simulink library "LibrarySimulinkSASv2016a"  (R2018a)
settings: Solver=ode45  StartTime=0.0  StopTime=10.0  RelTol=1e-3
undefined symbols (not defined in the file or in its folder): IdB, IdL, IdM, shmControl, ts
libraries: LibrarySimulinkSAS x15, $bdroot x1
(part 2 of 4)

== system LibrarySimulinkSASv2016a/Ardrone Parrot ==
  Pitch : Inport
  Roll : Inport  Port=2
  Gaz : Inport  Port=3
  Yaw rate : Inport  Port=4
  Generic Shared Memory Block : S-Function  FunctionName=matlabGenericSHM  Parameters=nombre,dispositivo,vecE,vecS,tipoE,tipoS,ts  SFunctionDeploymentMode=off  EnableBusSupport=off  mask(Generic Share Memory block V2.0){nombre=Dev, dispositivo=1, vecE=[ 1 1 1 1], vecS=[20], tipoE=['d' 'd' 'd'  'd' ], tipoS=['d'], ts=0}
      mask prompts: nombre "Share Memory base name"; dispositivo "Device Number"; vecE "Sizes input vector"; vecS "Sizes output vector"; tipoE "Data Types Inputs"; tipoS "Data Types Outputs"; ts "Sample Time"
      mask help:
      | This block allows Simulink communication with external processes through shared memory. It works on Unix and Windows.
      | More information in Help.
      mask Help:
      | Share Memory base name (nombre): It's the base string of share memory ej 'robot'
      | Device Number (dispositivo): integer that identifies the device between multiple in use
      | Two shared memorys are created with names:
      | "%name_entrada_%dispositivo" for the device input (writing)
      | "%name_salida_%dispositivo" for the device output (reading)
      | Sizes input vector (vecE)  : array that specify the number of inputs (array dimension) and the inputs size (array values)
      | Sizes output vector  (vecS): array that specify the number of outputs (array dimension) and the outputs size (array values)
      | Data types input(tipoE): char array that specify each input types
      | Data types output (tipoS): char array that specify each output types
      | Allowed types are:
      | ('f'=float, 'd'=double, 'i'=int32, 'c'=char, 'C'= unsigned char, 'I'=unsigned int)
      | Example.
      | Suppose the following parameters:
      | nombre='exampleMemory'
      | dispositivo=10
      | vecE=[3 2 1]
      | vecS=[6 5 7 8]
      | tipoE=['f' 'I' 'c']
      | tipoS=['C' 'i' 'd' 'd']
      | 
      | Two Share Memories are created with names:
      | "exampleMemory_entrada_10" (block input)
      | "exampleMemory_salida_10"  (block output)
      | 3 inputs are created:
      |  1° Dimension 3, type "unsigned char"
      |  2° Dimension 2, type "int"
      |  3° Dimension 1, type "char"
      | 4 outputs are created:
      |  1° Dimension 6, type "float"
      |  2° Dimension 5, type "unsigned int"
      |  3° Dimension 7, type "double"
      |  3° Dimension 8, type "double"
      mask Display: image('shmem.jpg')
  Simple Share Memory Block : S-Function  FunctionName=simpleSHM  Parameters=nombre,dispositivo,vecE,vecS,tipoE,tipoS  SFunctionDeploymentMode=off  EnableBusSupport=off  mask(Share Memory Block version 2.0){nombre='ARDRONE_video', dispositivo=1, vecE=[], vecS=[320*240*3], tipoE=[], tipoS=['C']}
      mask prompts: nombre "Share Memory base name"; dispositivo "Device Number"; vecE "Sizes input vector"; vecS "Sizes output vector"; tipoE "Data Types Inputs"; tipoS "Data Types Outputs"
      mask help: This block allows Simulink communication with external processes through shared memory. It works on Unix and Windows
      mask Help:
      | Share Memory base name (nombre): It's the base string of share memory ej 'robot'
      | Device Number (dispositivo): integer that identifies the device between multiple in use
      | Only one Share memory will be created, so you should specify only the inputs or outputs but not both
      | One shared memorys is created with name: "%name_%dispositivo"
      | 
      | Sizes input vector (vecE)  : array that specify the number of inputs (array dimension) and the inputs size (array values)
      | Sizes output vector  (vecS): array that specify the number of outputs (array dimension) and the outputs size (array values)
      | Data types input(tipoE): char array that specify each input types
      | Data types output (tipoS): char array that specify each output types
      | Allowed types are:
      | ('f'=float, 'd'=double, 'i'=int32, 'c'=char, 'C'= unsigned char, 'I'=unsigned int)
      | 
      | Example.
      | Suppose the following parameters:
      | nombre='exampleMemory'
      | dispositivo=10
      | vecE=[3 2 1]
      | vecS=[]
      | tipoE=['f' 'I' 'c']
      | tipoS=[]
      | 
      | One Share Memorie is created with name:
      | "exampleMemory_10" (block input)
      | 
      | 3 inputs are created:
      |  1° Dimension 3, type "unsigned char"
      |  2° Dimension 2, type "int"
      |  3° Dimension 1, type "char"
      mask Display: image('memoryChip.png')
  toRGB image1 : Subsystem  mask{ResX=320, ResY=240}
      mask prompts: ResX "Resolucion Camara X"; ResY "Resolucion Camara Y"
      mask Display:
      | port_label('output', 1, 'RGB')
      | port_label('input', 1, 'videoIN')
  Ardrone State : Outport
  Ardrone Video : Outport  Port=2
  wires:
  Generic Shared Memory Block/1 -> Ardrone State/1
  Yaw rate/1 -> Generic Shared Memory Block/4
  Gaz/1 -> Generic Shared Memory Block/3
  Pitch/1 -> Generic Shared Memory Block/1
  Roll/1 -> Generic Shared Memory Block/2
  toRGB image1/1(Out1) -> Ardrone Video/1
  Simple Share Memory Block/1 -> toRGB image1/1(In1)

== system LibrarySimulinkSASv2016a/Arduino+motor shield ==
  Run : Inport
  U1 : Inport  Port=2
  U2 : Inport  Port=3
  Generic Shared Memory Block : S-Function  FunctionName=matlabGenericSHM  Parameters=nombre,dispositivo,vecE,vecS,tipoE,tipoS,ts  SFunctionDeploymentMode=off  EnableBusSupport=off  mask(Generic Share Memory block V2.0){nombre='ARDUINO', dispositivo=dev, vecE=[1 1 1 ], vecS=[1 1 1 1 1 1 1 1 1], tipoE=['f' 'f' 'f' ], tipoS=['f' 'f' 'f' 'f' 'f' 'f' 'f' 'f' 'f'], ts=0}
      mask prompts: nombre "Share Memory base name"; dispositivo "Device Number"; vecE "Sizes input vector"; vecS "Sizes output vector"; tipoE "Data Types Inputs"; tipoS "Data Types Outputs"; ts "Sample Time"
      mask help:
      | This block allows Simulink communication with external processes through shared memory. It works on Unix and Windows.
      | More information in Help.
      mask Help:
      | Share Memory base name (nombre): It's the base string of share memory ej 'robot'
      | Device Number (dispositivo): integer that identifies the device between multiple in use
      | Two shared memorys are created with names:
      | "%name_entrada_%dispositivo" for the device input (writing)
      | "%name_salida_%dispositivo" for the device output (reading)
      | Sizes input vector (vecE)  : array that specify the number of inputs (array dimension) and the inputs size (array values)
      | Sizes output vector  (vecS): array that specify the number of outputs (array dimension) and the outputs size (array values)
      | Data types input(tipoE): char array that specify each input types
      | Data types output (tipoS): char array that specify each output types
      | Allowed types are:
      | ('f'=float, 'd'=double, 'i'=int32, 'c'=char, 'C'= unsigned char, 'I'=unsigned int)
      | Example.
      | Suppose the following parameters:
      | nombre='exampleMemory'
      | dispositivo=10
      | vecE=[3 2 1]
      | vecS=[6 5 7 8]
      | tipoE=['f' 'I' 'c']
      | tipoS=['C' 'i' 'd' 'd']
      | 
      | Two Share Memories are created with names:
      | "exampleMemory_entrada_10" (block input)
      | "exampleMemory_salida_10"  (block output)
      | 3 inputs are created:
      |  1° Dimension 3, type "unsigned char"
      |  2° Dimension 2, type "int"
      |  3° Dimension 1, type "char"
      | 4 outputs are created:
      |  1° Dimension 6, type "float"
      |  2° Dimension 5, type "unsigned int"
      |  3° Dimension 7, type "double"
      |  3° Dimension 8, type "double"
      mask Display: image('shmem.jpg')
  State : Outport
  AD0 : Outport  Port=2
  AD1 : Outport  Port=3
  AD2 : Outport  Port=4
  AD3 : Outport  Port=5
  AD4 : Outport  Port=6
  AD5 : Outport  Port=7
  AD6 : Outport  Port=8
  AD7 : Outport  Port=9
  wires:
  Run/1 -> Generic Shared Memory Block/1
  U1/1 -> Generic Shared Memory Block/2
  U2/1 -> Generic Shared Memory Block/3
  Generic Shared Memory Block/1 -> State/1
  Generic Shared Memory Block/2 -> AD0/1
  Generic Shared Memory Block/3 -> AD1/1
  Generic Shared Memory Block/4 -> AD2/1
  Generic Shared Memory Block/5 -> AD3/1
  Generic Shared Memory Block/6 -> AD4/1
  Generic Shared Memory Block/7 -> AD5/1
  Generic Shared Memory Block/8 -> AD6/1
  Generic Shared Memory Block/9 -> AD7/1

== system LibrarySimulinkSASv2016a/BOSCH SR-800 ==
  vel : Inport
  Constant : Constant  Value=[0;0]
  Demux : Demux
  Generic Share Memory Block V2.0 : S-Function  FunctionName=matlabGenericSHM  Parameters=nombre,dispositivo,vecE,vecS,tipoE,tipoS  SFunctionDeploymentMode=off  EnableBusSupport=off  mask(Generic Share Memory block V2.0){nombre='boschShareMemory', dispositivo=id, vecE=[4 ], vecS=[4], tipoE=['d' ], tipoS=['d']}
      mask prompts: nombre "Share Memory base name"; dispositivo "Device Number"; vecE "Sizes input vector"; vecS "Sizes output vector"; tipoE "Data Types Inputs"; tipoS "Data Types Outputs"
      mask help:
      | This block allows Simulink communication with external processes through shared memory. It works on Unix and Windows.
      | More information in Help.
      mask Help:
      | Share Memory base name (nombre): It's the base string of share memory ej 'robot'
      | Device Number (dispositivo): integer that identifies the device between multiple in use
      | Two shared memorys are created with names:
      | "%name_entrada_%dispositivo" for the device input (writing)
      | "%name_salida_%dispositivo" for the device output (reading)
      | Sizes input vector (vecE)  : array that specify the number of inputs (array dimension) and the inputs size (array values)
      | Sizes output vector  (vecS): array that specify the number of outputs (array dimension) and the outputs size (array values)
      | Data types input(tipoE): char array that specify each input types
      | Data types output (tipoS): char array that specify each output types
      | Allowed types are:
      | ('f'=float, 'd'=double, 'i'=int32, 'c'=char, 'C'= unsigned char, 'I'=unsigned int)
      | Example.
      | Suppose the following parameters:
      | nombre='exampleMemory'
      | dispositivo=10
      | vecE=[3 2 1]
      | vecS=[6 5 7 8]
      | tipoE=['f' 'I' 'c']
      | tipoS=['C' 'i' 'd' 'd']
      | 
      | Two Share Memories are created with names:
      | "exampleMemory_entrada_10" (block input)
      | "exampleMemory_salida_10"  (block output)
      | 3 inputs are created:
      |  1° Dimension 3, type "unsigned char"
      |  2° Dimension 2, type "int"
      |  3° Dimension 1, type "char"
      | 4 outputs are created:
      |  1° Dimension 6, type "float"
      |  2° Dimension 5, type "unsigned int"
      |  3° Dimension 7, type "double"
      |  3° Dimension 8, type "double"
      mask Display: image('shmem.jpg')
  Mux : Mux  Inputs=2
  Mux1 : Mux  Inputs=2
  Saturation : Saturate
  Terminator : Terminator
  Terminator1 : Terminator
  q : Outport
  wires:
  vel/1 -> Saturation/1
  Constant/1 -> Mux/2
  Mux/1 -> Generic Share Memory Block V2.0/1
  Generic Share Memory Block V2.0/1 -> Demux/1
  Demux/3 -> Terminator/1
  Demux/4 -> Terminator1/1
  Saturation/1 -> Mux/1
  Mux1/1 -> q/1
  Demux/1 -> Mux1/1
  Demux/2 -> Mux1/2

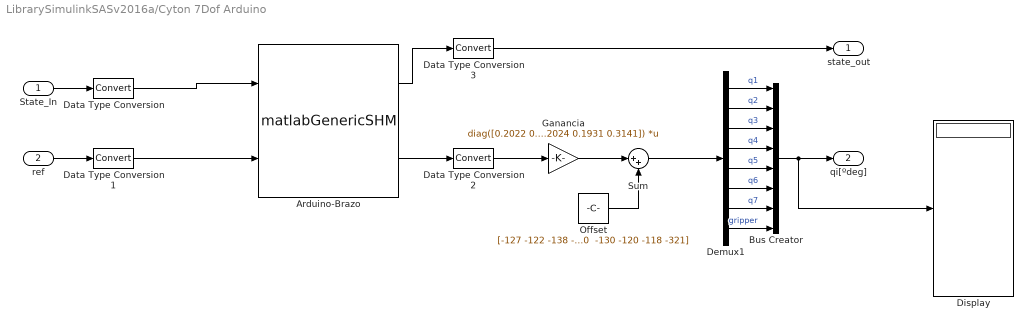
== system LibrarySimulinkSASv2016a/Cyton 7Dof Arduino ==
  State_In : Inport
  ref : Inport  Port=2
  Arduino-Brazo : S-Function  FunctionName=matlabGenericSHM  Parameters=nombre,dispositivo,vecE,vecS,tipoE,tipoS,ts  SFunctionDeploymentMode=off  EnableBusSupport=off  mask(Generic Share Memory block V2.0){nombre='ARDUINO', dispositivo=id, vecE=[1 8], vecS=[1 8], tipoE=['f' 'f'], tipoS=['f' 'f'], ts=ts}
      mask prompts: nombre "Share Memory base name"; dispositivo "Device Number"; vecE "Sizes input vector"; vecS "Sizes output vector"; tipoE "Data Types Inputs"; tipoS "Data Types Outputs"; ts "Sample Time"
      mask help:
      | This block allows Simulink communication with external processes through shared memory. It works on Unix and Windows.
      | More information in Help.
      mask Help:
      | Share Memory base name (nombre): It's the base string of share memory ej 'robot'
      | Device Number (dispositivo): integer that identifies the device between multiple in use
      | Two shared memorys are created with names:
      | "%name_entrada_%dispositivo" for the device input (writing)
      | "%name_salida_%dispositivo" for the device output (reading)
      | Sizes input vector (vecE)  : array that specify the number of inputs (array dimension) and the inputs size (array values)
      | Sizes output vector  (vecS): array that specify the number of outputs (array dimension) and the outputs size (array values)
      | Data types input(tipoE): char array that specify each input types
      | Data types output (tipoS): char array that specify each output types
      | Allowed types are:
      | ('f'=float, 'd'=double, 'i'=int32, 'c'=char, 'C'= unsigned char, 'I'=unsigned int)
      | Example.
      | Suppose the following parameters:
      | nombre='exampleMemory'
      | dispositivo=10
      | vecE=[3 2 1]
      | vecS=[6 5 7 8]
      | tipoE=['f' 'I' 'c']
      | tipoS=['C' 'i' 'd' 'd']
      | 
      | Two Share Memories are created with names:
      | "exampleMemory_entrada_10" (block input)
      | "exampleMemory_salida_10"  (block output)
      | 3 inputs are created:
      |  1° Dimension 3, type "unsigned char"
      |  2° Dimension 2, type "int"
      |  3° Dimension 1, type "char"
      | 4 outputs are created:
      |  1° Dimension 6, type "float"
      |  2° Dimension 5, type "unsigned int"
      |  3° Dimension 7, type "double"
      |  3° Dimension 8, type "double"
      mask Display: image('shmem.jpg')
  Bus Creator : BusCreator  Inputs=8
  Data Type Conversion{,1..3} (4 alike) : DataTypeConversion
  Demux1 : Demux  Outputs=8
  Display : Display
  Ganancia : Gain  Gain=diag([0.2022 0.2057 0.2093 0.2047 0.2179 0.2024 0.1931 0.3141])  Multiplication=Matrix(K*u) (u vector)
  Offset : Constant  Value="[-127 -122 -138 -110  -130 -120 -118 -321]"
  Sum : Sum  Inputs=|++
  state_out : Outport
  qi[ºdeg] : Outport  Port=2
  wires:
  Data Type Conversion3/1 -> state_out/1
  Data Type Conversion2/1 -> Ganancia/1
  Offset/1 -> Sum/2
  Sum/1 -> Demux1/1
  Ganancia/1 -> Sum/1
  Bus Creator/1 -> qi[ºdeg]/1, Display/1
  Demux1/1 -> Bus Creator/1  "q1"
  Demux1/2 -> Bus Creator/2  "q2"
  Demux1/3 -> Bus Creator/3  "q3"
  Demux1/4 -> Bus Creator/4  "q4"
  Demux1/5 -> Bus Creator/5  "q5"
  Demux1/6 -> Bus Creator/6  "q6"
  Demux1/7 -> Bus Creator/7  "q7"
  Demux1/8 -> Bus Creator/8  "gripper"
  ref/1 -> Data Type Conversion1/1
  Arduino-Brazo/2 -> Data Type Conversion2/1
  Arduino-Brazo/1 -> Data Type Conversion3/1
  State_In/1 -> Data Type Conversion/1
  Data Type Conversion/1 -> Arduino-Brazo/1
  Data Type Conversion1/1 -> Arduino-Brazo/2

== system LibrarySimulinkSASv2016a/HyperImu Client ==
  Parameter : Inport
  Generic Shared Memory Block : S-Function  FunctionName=matlabGenericSHM  Parameters=nombre,dispositivo,vecE,vecS,tipoE,tipoS,ts  SFunctionDeploymentMode=off  EnableBusSupport=off  mask(Generic Share Memory block V2.0){nombre='HYPERIMU', dispositivo=dev, vecE=[1], vecS=[12 1 1], tipoE=['f'], tipoS=['f' 'I' 'f'], ts=0}
      mask prompts: nombre "Share Memory base name"; dispositivo "Device Number"; vecE "Sizes input vector"; vecS "Sizes output vector"; tipoE "Data Types Inputs"; tipoS "Data Types Outputs"; ts "Sample Time"
      mask help:
      | This block allows Simulink communication with external processes through shared memory. It works on Unix and Windows.
      | More information in Help.
      mask Help:
      | Share Memory base name (nombre): It's the base string of share memory ej 'robot'
      | Device Number (dispositivo): integer that identifies the device between multiple in use
      | Two shared memorys are created with names:
      | "%name_entrada_%dispositivo" for the device input (writing)
      | "%name_salida_%dispositivo" for the device output (reading)
      | Sizes input vector (vecE)  : array that specify the number of inputs (array dimension) and the inputs size (array values)
      | Sizes output vector  (vecS): array that specify the number of outputs (array dimension) and the outputs size (array values)
      | Data types input(tipoE): char array that specify each input types
      | Data types output (tipoS): char array that specify each output types
      | Allowed types are:
      | ('f'=float, 'd'=double, 'i'=int32, 'c'=char, 'C'= unsigned char, 'I'=unsigned int)
      | Example.
      | Suppose the following parameters:
      | nombre='exampleMemory'
      | dispositivo=10
      | vecE=[3 2 1]
      | vecS=[6 5 7 8]
      | tipoE=['f' 'I' 'c']
      | tipoS=['C' 'i' 'd' 'd']
      | 
      | Two Share Memories are created with names:
      | "exampleMemory_entrada_10" (block input)
      | "exampleMemory_salida_10"  (block output)
      | 3 inputs are created:
      |  1° Dimension 3, type "unsigned char"
      |  2° Dimension 2, type "int"
      |  3° Dimension 1, type "char"
      | 4 outputs are created:
      |  1° Dimension 6, type "float"
      |  2° Dimension 5, type "unsigned int"
      |  3° Dimension 7, type "double"
      |  3° Dimension 8, type "double"
      mask Display: image('shmem.jpg')
  Estate HyperIMU : Outport
  Rx Packets Number : Outport  Port=2
  Interpacket gap : Outport  Port=3
  wires:
  Parameter/1 -> Generic Shared Memory Block/1
  Generic Shared Memory Block/1 -> Estate HyperIMU/1
  Generic Shared Memory Block/3 -> Interpacket gap/1
  Generic Shared Memory Block/2 -> Rx Packets Number/1

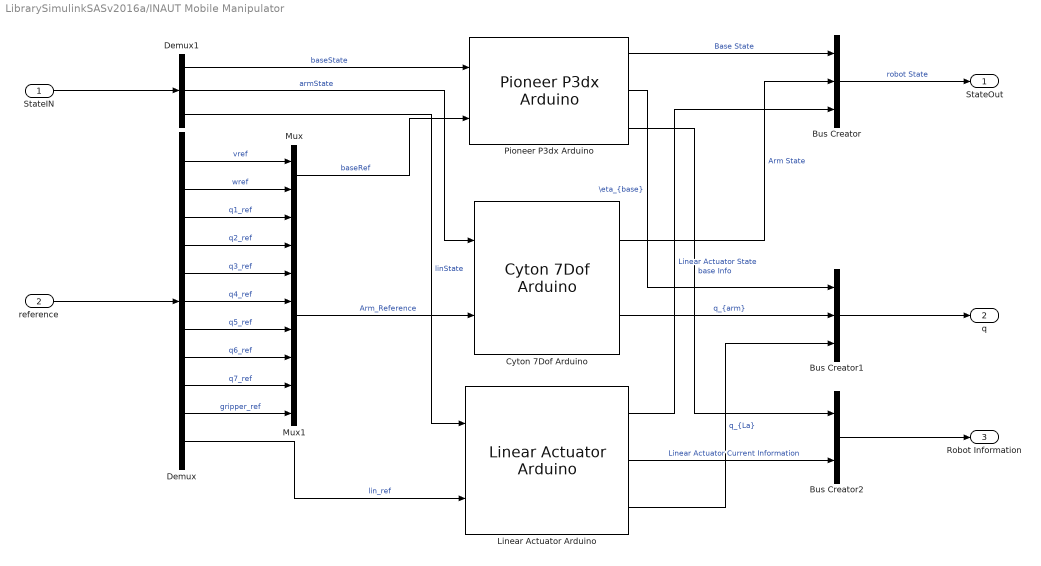
== system LibrarySimulinkSASv2016a/INAUT Mobile Manipulator ==
  StateIN : Inport
  reference : Inport  Port=2
  Bus Creator : BusCreator  Inputs=3
  Bus Creator1 : BusCreator  Inputs=3
  Bus Creator2 : BusCreator  Inputs=2
  Cyton 7Dof Arduino : LibrarySimulinkSAS/Cyton 7Dof Arduino  id=IdM  ts=ts
  Demux : Demux  Outputs=2+8+1
  Demux1 : Demux  Outputs=3
  Linear Actuator Arduino : LibrarySimulinkSAS/Linear Actuator Arduino  id=IdL  ts=ts
  Mux : Mux  Inputs=2
  Mux1 : Mux  Inputs=8
  Pioneer P3dx Arduino : LibrarySimulinkSAS/Pioneer P3dx Arduino  id=IdB  ts=ts
  StateOut : Outport
  q : Outport  Port=2
  Robot Information : Outport  Port=3
  wires:
  Bus Creator2/1 -> Robot Information/1
  Bus Creator1/1 -> q/1
  Pioneer P3dx Arduino/1 -> Bus Creator/1  "Base State"
  Cyton 7Dof Arduino/1 -> Bus Creator/2  "Arm State"
  Mux/1 -> Pioneer P3dx Arduino/2  "baseRef"
  Demux1/3 -> Linear Actuator Arduino/1  "linState"
  Demux1/2 -> Cyton 7Dof Arduino/1  "armState"
  Demux1/1 -> Pioneer P3dx Arduino/1  "baseState"
  Bus Creator/1 -> StateOut/1  "robot State"
  Demux/11 -> Linear Actuator Arduino/2  "lin_ref"
  Mux1/1 -> Cyton 7Dof Arduino/2  "Arm_Reference"
  Demux/10 -> Mux1/8  "gripper_ref"
  Demux/9 -> Mux1/7  "q7_ref"
  Demux/8 -> Mux1/6  "q6_ref"
  Demux/7 -> Mux1/5  "q5_ref"
  Demux/6 -> Mux1/4  "q4_ref"
  Demux/5 -> Mux1/3  "q3_ref"
  Demux/4 -> Mux1/2  "q2_ref"
  Demux/3 -> Mux1/1  "q1_ref"
  Demux/2 -> Mux/2  "wref"
  Demux/1 -> Mux/1  "vref"
  Pioneer P3dx Arduino/2 -> Bus Creator1/1  "\\eta_{base}"
  Pioneer P3dx Arduino/3 -> Bus Creator2/1  "base Info"
  Cyton 7Dof Arduino/2 -> Bus Creator1/2  "q_{arm}"
  Linear Actuator Arduino/3 -> Bus Creator1/3  "q_{La}"
  Linear Actuator Arduino/2 -> Bus Creator2/2  "Linear Actuator Current Information"
  Linear Actuator Arduino/1 -> Bus Creator/3  "Linear Actuator State"
  StateIN/1 -> Demux1/1
  reference/1 -> Demux/1

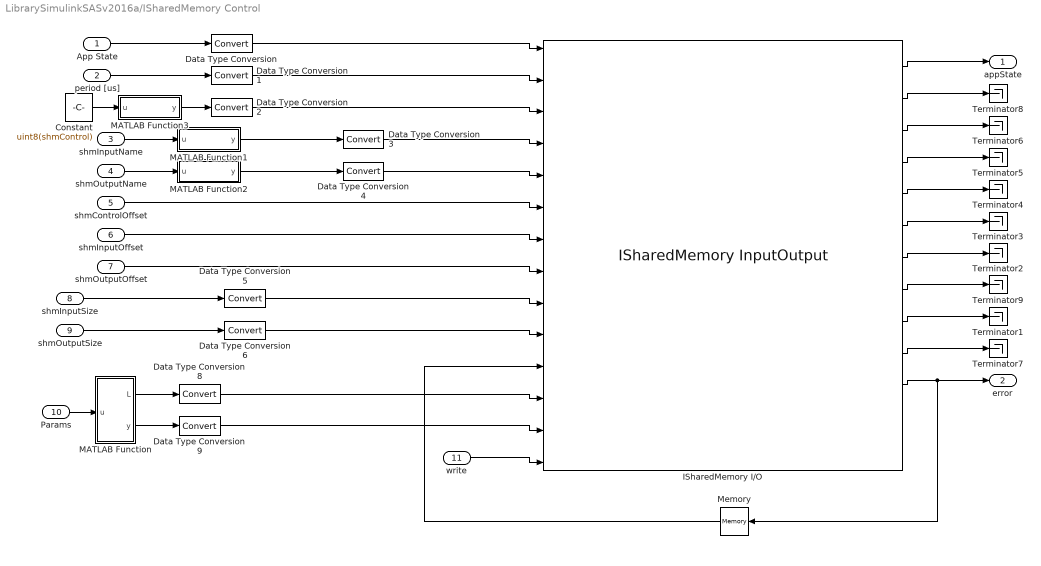
== system LibrarySimulinkSASv2016a/ISharedMemory Control ==
  App State : Inport
  period [us] : Inport  Port=2
  shmInputName : Inport  Port=3
  shmOutputName : Inport  Port=4
  shmControlOffset : Inport  Port=5
  shmInputOffset : Inport  Port=6
  shmOutputOffset : Inport  Port=7
  shmInputSize : Inport  Port=8
  shmOutputSize : Inport  Port=9
  Params : Inport  Port=10
  write : Inport  Port=11
  Constant : Constant  Value=uint8(shmControl)
  Data Type Conversion{,1..6,8..9} (9 alike) : DataTypeConversion
  ISharedMemory I/O : LibrarySimulinkSAS/ISharedMemory InputOutput  Priority=10  nameIn=shmControl  nameOut=shmControl  vecE=[1 1 40 40 40 1 1 1 1 1 1 1 400]  vecS=[1 1 40 40 40 1 1 1 1 1 1 1 400]  tipoE=['i' 'i' 'c' 'c' 'c' 'i' 'i' 'i' 'i' 'i' 'i' 'i' 'c']  tipoS=['i' 'i' 'c' 'c' 'c' 'i' 'i' 'i' 'i' 'i' 'i' 'i' 'c']  inOff=0  outOff=0  ts=-1  trigg=on
  MATLAB Function : MATLAB Function  TreatAsAtomicUnit=on
  MATLAB Function1 : MATLAB Function  TreatAsAtomicUnit=on
  MATLAB Function2 : MATLAB Function  TreatAsAtomicUnit=on
  MATLAB Function3 : MATLAB Function  TreatAsAtomicUnit=on
  Memory : Memory
  Terminator{1..9} (9 alike) : Terminator
  appState : Outport
  error : Outport  Port=2
  wires:
  Constant/1 -> MATLAB Function3/1(u)
  shmOutputOffset/1 -> ISharedMemory I/O/8
  shmInputOffset/1 -> ISharedMemory I/O/7
  shmControlOffset/1 -> ISharedMemory I/O/6
  Data Type Conversion3/1 -> ISharedMemory I/O/4
  Data Type Conversion4/1 -> ISharedMemory I/O/5
  Data Type Conversion2/1 -> ISharedMemory I/O/3
  Data Type Conversion1/1 -> ISharedMemory I/O/2
  Data Type Conversion/1 -> ISharedMemory I/O/1
  Data Type Conversion5/1 -> ISharedMemory I/O/9
  Data Type Conversion6/1 -> ISharedMemory I/O/10
  ISharedMemory I/O/8 -> Terminator9/1
  ISharedMemory I/O/11 -> Memory/1, error/1
  Data Type Conversion8/1 -> ISharedMemory I/O/12
  Data Type Conversion9/1 -> ISharedMemory I/O/13
  write/1 -> ISharedMemory I/O/14
  period [us]/1 -> Data Type Conversion1/1
  App State/1 -> Data Type Conversion/1
  Params/1 -> MATLAB Function/1(u)
  shmOutputSize/1 -> Data Type Conversion6/1
  shmOutputName/1 -> MATLAB Function2/1(u)
  shmInputSize/1 -> Data Type Conversion5/1
  shmInputName/1 -> MATLAB Function1/1(u)
  MATLAB Function/2(y) -> Data Type Conversion9/1
  MATLAB Function1/1(y) -> Data Type Conversion3/1
  MATLAB Function2/1(y) -> Data Type Conversion4/1
  MATLAB Function3/1(y) -> Data Type Conversion2/1
  MATLAB Function/1(L) -> Data Type Conversion8/1
  ISharedMemory I/O/1 -> appState/1
  Memory/1 -> ISharedMemory I/O/11
  ISharedMemory I/O/10 -> Terminator7/1
  ISharedMemory I/O/9 -> Terminator1/1
  ISharedMemory I/O/7 -> Terminator2/1
  ISharedMemory I/O/6 -> Terminator3/1
  ISharedMemory I/O/5 -> Terminator4/1
  ISharedMemory I/O/4 -> Terminator5/1
  ISharedMemory I/O/3 -> Terminator6/1
  ISharedMemory I/O/2 -> Terminator8/1

== system LibrarySimulinkSASv2016a/Linear Actuator Arduino ==
  State_in : Inport
  reference[mm] : Inport  Port=2
  Arduino-Actuador : S-Function  FunctionName=matlabGenericSHM  Parameters=nombre,dispositivo,vecE,vecS,tipoE,tipoS,ts  SFunctionDeploymentMode=off  EnableBusSupport=off  mask(Generic Share Memory block V2.0){nombre='ARDUINO', dispositivo=id, vecE=[1 1], vecS=[1 3 1], tipoE=['f' 'f'], tipoS=['f' 'f' 'f'], ts=ts}
      mask prompts: nombre "Share Memory base name"; dispositivo "Device Number"; vecE "Sizes input vector"; vecS "Sizes output vector"; tipoE "Data Types Inputs"; tipoS "Data Types Outputs"; ts "Sample Time"
      mask help:
      | This block allows Simulink communication with external processes through shared memory. It works on Unix and Windows.
      | More information in Help.
      mask Help:
      | Share Memory base name (nombre): It's the base string of share memory ej 'robot'
      | Device Number (dispositivo): integer that identifies the device between multiple in use
      | Two shared memorys are created with names:
      | "%name_entrada_%dispositivo" for the device input (writing)
      | "%name_salida_%dispositivo" for the device output (reading)
      | Sizes input vector (vecE)  : array that specify the number of inputs (array dimension) and the inputs size (array values)
      | Sizes output vector  (vecS): array that specify the number of outputs (array dimension) and the outputs size (array values)
      | Data types input(tipoE): char array that specify each input types
      | Data types output (tipoS): char array that specify each output types
      | Allowed types are:
      | ('f'=float, 'd'=double, 'i'=int32, 'c'=char, 'C'= unsigned char, 'I'=unsigned int)
      | Example.
      | Suppose the following parameters:
      | nombre='exampleMemory'
      | dispositivo=10
      | vecE=[3 2 1]
      | vecS=[6 5 7 8]
      | tipoE=['f' 'I' 'c']
      | tipoS=['C' 'i' 'd' 'd']
      | 
      | Two Share Memories are created with names:
      | "exampleMemory_entrada_10" (block input)
      | "exampleMemory_salida_10"  (block output)
      | 3 inputs are created:
      |  1° Dimension 3, type "unsigned char"
      |  2° Dimension 2, type "int"
      |  3° Dimension 1, type "char"
      | 4 outputs are created:
      |  1° Dimension 6, type "float"
      |  2° Dimension 5, type "unsigned int"
      |  3° Dimension 7, type "double"
      |  3° Dimension 8, type "double"
      mask Display: image('shmem.jpg')
  Bus Creator : BusCreator  Inputs=3
  Data Type Conversion{,1..3,6} (5 alike) : DataTypeConversion
  Demux : Demux  Outputs=3
  Sate_out : Outport
  Current sensors[mA] : Outport  Port=2
  Position[mm] : Outport  Port=3
  wires:
  Data Type Conversion3/1 -> Demux/1
  Arduino-Actuador/2 -> Data Type Conversion3/1  "Linear Actuator Current Sensor 1"
  Data Type Conversion6/1 -> Position[mm]/1
  Data Type Conversion2/1 -> Sate_out/1
  Bus Creator/1 -> Current sensors[mA]/1
  Demux/1 -> Bus Creator/1  "Linear Actuator Current Sensor 1"
  Demux/2 -> Bus Creator/2  "Linear Actuator Current Sensor 2"
  Demux/3 -> Bus Creator/3  "Linear Actuator Current Sensor 3"
  Arduino-Actuador/1 -> Data Type Conversion2/1
  State_in/1 -> Data Type Conversion/1
  Arduino-Actuador/3 -> Data Type Conversion6/1
  reference[mm]/1 -> Data Type Conversion1/1
  Data Type Conversion1/1 -> Arduino-Actuador/2
  Data Type Conversion/1 -> Arduino-Actuador/1

== system LibrarySimulinkSASv2016a/Neurosky ==
  In1 : Inport
  Generic Shared Memory Block : S-Function  FunctionName=matlabGenericSHM  Parameters=nombre,dispositivo,vecE,vecS,tipoE,tipoS,ts  SFunctionDeploymentMode=off  EnableBusSupport=off  mask(Generic Share Memory block V2.0){nombre='NEUROSKY', dispositivo=id, vecE=[1], vecS=[1 1 1 1 1 1 1 1 1 1 1 1], tipoE=['f'], tipoS=['f' 'f' 'f' 'f' 'f' 'f' 'f' 'f' 'f' 'f' 'f' 'f'], ts=ts}
      mask prompts: nombre "Share Memory base name"; dispositivo "Device Number"; vecE "Sizes input vector"; vecS "Sizes output vector"; tipoE "Data Types Inputs"; tipoS "Data Types Outputs"; ts "Sample time"
      mask help:
      | This block allows Simulink communication with external processes through shared memory. It works on Unix and Windows.
      | More information in Help.
      mask Help:
      | Share Memory base name (nombre): It's the base string of share memory ej 'robot'
      | Device Number (dispositivo): integer that identifies the device between multiple in use
      | Two shared memorys are created with names:
      | "%name_entrada_%dispositivo" for the device input (writing)
      | "%name_salida_%dispositivo" for the device output (reading)
      | Sizes input vector (vecE)  : array that specify the number of inputs (array dimension) and the inputs size (array values)
      | Sizes output vector  (vecS): array that specify the number of outputs (array dimension) and the outputs size (array values)
      | Data types input(tipoE): char array that specify each input types
      | Data types output (tipoS): char array that specify each output types
      | Allowed types are:
      | ('f'=float, 'd'=double, 'i'=int32, 'c'=char, 'C'= unsigned char, 'I'=unsigned int)
      | Example.
      | Suppose the following parameters:
      | nombre='exampleMemory'
      | dispositivo=10
      | vecE=[3 2 1]
      | vecS=[6 5 7 8]
      | tipoE=['f' 'I' 'c']
      | tipoS=['C' 'i' 'd' 'd']
      | 
      | Two Share Memories are created with names:
      | "exampleMemory_entrada_10" (block input)
      | "exampleMemory_salida_10"  (block output)
      | 3 inputs are created:
      |  1° Dimension 3, type "unsigned char"
      |  2° Dimension 2, type "int"
      |  3° Dimension 1, type "char"
      | 4 outputs are created:
      |  1° Dimension 6, type "float"
      |  2° Dimension 5, type "unsigned int"
      |  3° Dimension 7, type "double"
      |  3° Dimension 8, type "double"
      mask Display: image('shmem.jpg')
  Out1 : Outport
  Out2 : Outport  Port=2
  Out3 : Outport  Port=3
  Out4 : Outport  Port=4
  Out5 : Outport  Port=5
  Out6 : Outport  Port=6
  Out7 : Outport  Port=7
  Out8 : Outport  Port=8
  Out9 : Outport  Port=9
  Out10 : Outport  Port=10
  Out11 : Outport  Port=11
  Out12 : Outport  Port=12
  wires:
  Generic Shared Memory Block/3 -> Out3/1
  Generic Shared Memory Block/7 -> Out7/1
  Generic Shared Memory Block/9 -> Out9/1
  Generic Shared Memory Block/5 -> Out5/1
  Generic Shared Memory Block/8 -> Out8/1
  Generic Shared Memory Block/2 -> Out2/1
  Generic Shared Memory Block/11 -> Out11/1
  Generic Shared Memory Block/12 -> Out12/1
  Generic Shared Memory Block/10 -> Out10/1
  Generic Shared Memory Block/4 -> Out4/1
  Generic Shared Memory Block/6 -> Out6/1
  Generic Shared Memory Block/1 -> Out1/1
  In1/1 -> Generic Shared Memory Block/1

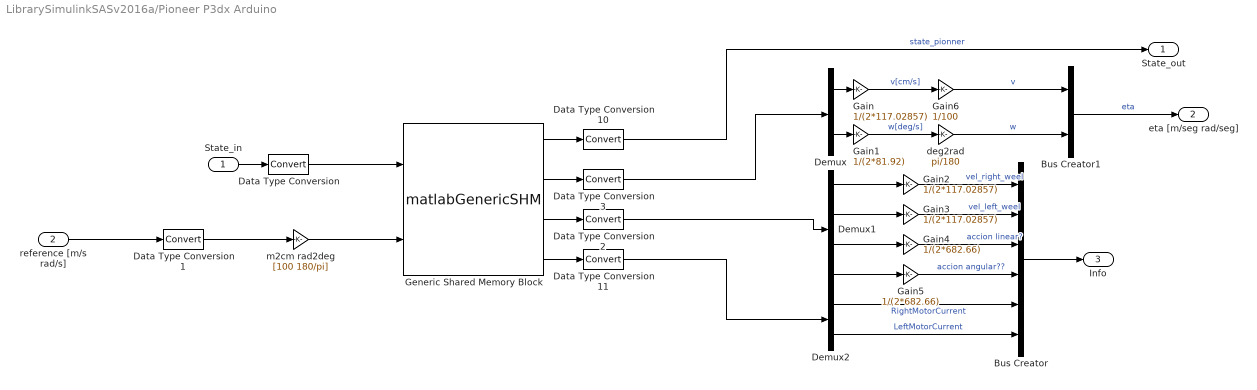
== system LibrarySimulinkSASv2016a/Pioneer P3dx Arduino ==
  State_in : Inport
  reference [m/s rad/s] : Inport  Port=2
  Bus Creator : BusCreator  Inputs=6
  Bus Creator1 : BusCreator  Inputs=2
  Data Type Conversion{,1..3,10..11} (6 alike) : DataTypeConversion
  Demux : Demux  Outputs=2
  Demux1 : Demux
  Demux2 : Demux  Outputs=2
  Gain : Gain  Gain=1/(2*117.02857)
  Gain1 : Gain  Gain=1/(2*81.92)
  Gain2 : Gain  Gain=1/(2*117.02857)
  Gain3 : Gain  Gain=1/(2*117.02857)
  Gain4 : Gain  Gain=1/(2*682.66)
  Gain5 : Gain  Gain=1/(2*682.66)
  Gain6 : Gain  Gain=1/100
  Generic Shared Memory Block : S-Function  FunctionName=matlabGenericSHM  Parameters=nombre,dispositivo,vecE,vecS,tipoE,tipoS,ts  SFunctionDeploymentMode=off  EnableBusSupport=off  mask(Generic Share Memory block V2.0){nombre='ARDUINO', dispositivo=id, vecE=[1 2], vecS=[1 2 4 2], tipoE=['f' 'f'], tipoS=['f' 'f' 'f' 'f' ], ts=ts}
      mask prompts: nombre "Share Memory base name"; dispositivo "Device Number"; vecE "Sizes input vector"; vecS "Sizes output vector"; tipoE "Data Types Inputs"; tipoS "Data Types Outputs"; ts "Sample Time"
      mask help:
      | This block allows Simulink communication with external processes through shared memory. It works on Unix and Windows.
      | More information in Help.
      mask Help:
      | Share Memory base name (nombre): It's the base string of share memory ej 'robot'
      | Device Number (dispositivo): integer that identifies the device between multiple in use
      | Two shared memorys are created with names:
      | "%name_entrada_%dispositivo" for the device input (writing)
      | "%name_salida_%dispositivo" for the device output (reading)
      | Sizes input vector (vecE)  : array that specify the number of inputs (array dimension) and the inputs size (array values)
      | Sizes output vector  (vecS): array that specify the number of outputs (array dimension) and the outputs size (array values)
      | Data types input(tipoE): char array that specify each input types
      | Data types output (tipoS): char array that specify each output types
      | Allowed types are:
      | ('f'=float, 'd'=double, 'i'=int32, 'c'=char, 'C'= unsigned char, 'I'=unsigned int)
      | Example.
      | Suppose the following parameters:
      | nombre='exampleMemory'
      | dispositivo=10
      | vecE=[3 2 1]
      | vecS=[6 5 7 8]
      | tipoE=['f' 'I' 'c']
      | tipoS=['C' 'i' 'd' 'd']
      | 
      | Two Share Memories are created with names:
      | "exampleMemory_entrada_10" (block input)
      | "exampleMemory_salida_10"  (block output)
      | 3 inputs are created:
      |  1° Dimension 3, type "unsigned char"
      |  2° Dimension 2, type "int"
      |  3° Dimension 1, type "char"
      | 4 outputs are created:
      |  1° Dimension 6, type "float"
      |  2° Dimension 5, type "unsigned int"
      |  3° Dimension 7, type "double"
      |  3° Dimension 8, type "double"
      mask Display: image('shmem.jpg')
  deg2rad : Gain  Gain=pi/180
  m2cm rad2deg : Gain  Gain=[100 180/pi]
  State_out : Outport
  eta [m/seg rad/seg] : Outport  Port=2
  Info : Outport  Port=3
  wires:
  Gain1/1 -> deg2rad/1  "w[deg/s]"
  Gain/1 -> Gain6/1  "v[cm/s]"
  deg2rad/1 -> Bus Creator1/2  "w"
  Gain6/1 -> Bus Creator1/1  "v"
  Data Type Conversion11/1 -> Demux2/1
  Data Type Conversion2/1 -> Demux1/1
  Data Type Conversion3/1 -> Demux/1
  Generic Shared Memory Block/2 -> Data Type Conversion3/1
  Generic Shared Memory Block/3 -> Data Type Conversion2/1
  Generic Shared Memory Block/4 -> Data Type Conversion11/1
  Generic Shared Memory Block/1 -> Data Type Conversion10/1
  m2cm rad2deg/1 -> Generic Shared Memory Block/2
  Demux2/2 -> Bus Creator/6  "LeftMotorCurrent"
  Demux2/1 -> Bus Creator/5  "RightMotorCurrent"
  Demux1/4 -> Gain5/1
  Demux1/3 -> Gain4/1
  Demux1/2 -> Gain3/1
  Demux1/1 -> Gain2/1
  Demux/2 -> Gain1/1
  Demux/1 -> Gain/1
  Data Type Conversion10/1 -> State_out/1  "state_pionner"
  Bus Creator1/1 -> eta [m/seg rad/seg]/1  "eta"
  Gain2/1 -> Bus Creator/1  "vel_right_weel"
  Gain5/1 -> Bus Creator/4  "accion angular??"
  Gain4/1 -> Bus Creator/3  "accion linear?"
  Gain3/1 -> Bus Creator/2  "vel_left_weel"
  Bus Creator/1 -> Info/1
  reference [m/s rad/s]/1 -> Data Type Conversion1/1
  State_in/1 -> Data Type Conversion/1
  Data Type Conversion1/1 -> m2cm rad2deg/1
  Data Type Conversion/1 -> Generic Shared Memory Block/1

== system LibrarySimulinkSASv2016a/Shared Memory Step synchronization ==
  MATLAB Function2 : MATLAB Function  TreatAsAtomicUnit=on
  Unit Delay : UnitDelay  SampleTime=-1
  Vrep-Count : S-Function  FunctionName=simpleSHM  Parameters=nombre,dispositivo,vecE,vecS,tipoE,tipoS  SFunctionDeploymentMode=off  EnableBusSupport=off  mask(Share Memory Block version 2.0){nombre='sync_counter', dispositivo=id, vecE=[], vecS=[1], tipoE=[], tipoS=['i']}
      mask prompts: nombre "Share Memory base name"; dispositivo "Device Number"; vecE "Sizes input vector"; vecS "Sizes output vector"; tipoE "Data Types Inputs"; tipoS "Data Types Outputs"
      mask help: This block allows Simulink communication with external processes through shared memory. It works on Unix and Windows
      mask Help:
      | Share Memory base name (nombre): It's the base string of share memory ej 'robot'
      | Device Number (dispositivo): integer that identifies the device between multiple in use
      | Only one Share memory will be created, so you should specify only the inputs or outputs but not both
      | One shared memorys is created with name: "%name_%dispositivo"
      | 
      | Sizes input vector (vecE)  : array that specify the number of inputs (array dimension) and the inputs size (array values)
      | Sizes output vector  (vecS): array that specify the number of outputs (array dimension) and the outputs size (array values)
      | Data types input(tipoE): char array that specify each input types
      | Data types output (tipoS): char array that specify each output types
      | Allowed types are:
      | ('f'=float, 'd'=double, 'i'=int32, 'c'=char, 'C'= unsigned char, 'I'=unsigned int)
      | 
      | Example.
      | Suppose the following parameters:
      | nombre='exampleMemory'
      | dispositivo=10
      | vecE=[3 2 1]
      | vecS=[]
      | tipoE=['f' 'I' 'c']
      | tipoS=[]
      | 
      | One Share Memorie is created with name:
      | "exampleMemory_10" (block input)
      | 
      | 3 inputs are created:
      |  1° Dimension 3, type "unsigned char"
      |  2° Dimension 2, type "int"
      |  3° Dimension 1, type "char"
      mask Display: image('memoryChip.png')
  triger : Outport
  wires:
  Vrep-Count/1 -> MATLAB Function2/1(cnt_0), Unit Delay/1
  Unit Delay/1 -> MATLAB Function2/2(cnt_1)
  MATLAB Function2/1(y) -> triger/1

== system LibrarySimulinkSASv2016a/UDP Data Client ==
  Data In : Inport
  ClientCom : S-Function  FunctionName=ClientCom  SFunctionDeploymentMode=off  EnableBusSupport=off
      mask Help:
      | HELP DEVICE
      | 
      | This block is used to transmit information over the network using TCP or UDP protocol.
      | This block is used to transmit small data vectors. For large sizes use the block 'ClientComVideo'.
      | 
      | -------------------------------------------------------------------------------------------------------------------------------------------------------
      | 
      | HELP DRIVERS
      | 
      | 
      | 
      | -------------------------------------------------------------------------------------------------------------------------------------------------------
      | HELP APP
      | 
      | This block depends on the external program 'Cliente.exe' placed on '\SAS TOOLS\Software Network Communication V2.0.0\Data Transmission\Cliente'
      | After executing, the program is started minimized in the taskbar.
      | To initiate communication first configure the program : right click on the icon ->Configurar.
      | On section 'Configuracion de Ip y Puertos', load the input IP address and the output IP address of the Client adaptor. If there are multiple adaptors, these IP may be identical or different for the input and output data. Also select the ports for input and output.
      | On section 'Datos de Conexion con el Servidor', load the IP address and the input port of the Server.
      | On seccion 'Protocolo' select TCP or UDP. (The TCP option still in development)
      | 
      | --------------------------------------------------------------------------------------------------------------------------------------------
      | 
      | HELP BLOCK
      | 
      | Input Ports
      | 
      | The vector data to be transmitted must be introduced into the port 'dataSend'. Its maximun size is 1024 doubles.
      | The size of data to be transmitted must be specified on port 'sizeSend'.
      | The transmission occurs in each pulse on port 'Clock'. Use this port to set the transmission rate.
      | 
      | Output Ports
      | 
      | Port 'stateChanel'contains information concerning the transmission of data. For details of the composition of this vector right-click the icon of the taskbar -> 'Estado'
      | Port 'dataRecv' contains the data vector received from the server.
      | 
      | ---------------------------------------------------------------------------------------------------------------------------------------------------------------------
      | 
      | HELP SHARE MEMORY
      | 
      | Shared Memory Structure:
      | 
      | typedef struct tag_NET_VIRTUAL_DEVICE_IN {
      | 	bool signalWrite;
      | 	UINT sizeMemoryOut;
      | } NET_VIRTUAL_DEVICE_IN;
      | 
      | typedef struct tag_NET_VIRTUAL_DEVICE_OUT {
      | 	bool signalRead;	//Se leen los flancos
      | 	UINT numberSentPackets;
      | 	UINT numberRecvPackets;
      | 	UINT numberLostPackets;
      | 	UINT numberDelPackets;
      | 	float rateSentPackets;
      | 	float rateRecvPackets;
      | 	float rateLostPackets;
      | 	float rateDelPackets;
      | 	float roundTripDelay;
      | 
      | 	UINT remoteNumberSentPackets;
      | 	UINT remoteNumberRecvPackets;
      | 	UINT remoteNumberLostPackets;
      | 	UINT remoteNumberDelPackets;
      | 	float remoteRateSentPackets;
      | 	float remoteRateRecvPackets;
      | 	float remoteRateLostPackets;
      | 	float remoteRateDelPackets;
      | 	float remoteRoundTripDelay;
      | } NET_VIRTUAL_DEVICE_OUT;
      | 
      | 
      | typedef struct tag_VIRTUAL_DEVICE_IN {
      | 	float dataMemory[1024];
      | } VIRTUAL_DEVICE_IN;
      | 
      | typedef struct tag_VIRTUAL_DEVICE_OUT {
      | 	float dataMemory[1024];
      | } VIRTUAL_DEVICE_OUT;
      | 
      | 
      | -------------------------------------------------------------------------------------------------------------------------------------------
      mask DialogControl DescGroupVar Prompt: Client video transmission through the network
      mask DialogControl DescTextVar Prompt: This block is used to exchange small amount of data over a network. Use this block client-side communication.
      mask Display:
      | image('client.png')
      | color('red')
      | port_label('input',1,'clock')
      | port_label('input',2,'sizeSend')
      | port_label('input',3,'dataSend')
      | color('blue')
      | port_label('output',1,'stateChannel')
      | port_label('output',2,'dataRecv')
  MATLAB Function : MATLAB Function  TreatAsAtomicUnit=on
  Selector : Selector  InputPortWidth=1024  IndexOptions=Starting index (dialog)  Indices=1  OutputSizes=n
  Signal Generator : SignalGenerator  WaveForm=square  Frequency=1/ts
  Chanel State : Outport
  Data out : Outport  Port=2
  wires:
  ClientCom/2 -> Selector/1
  Selector/1 -> Data out/1
  Data In/1 -> MATLAB Function/1(data)
  Signal Generator/1 -> ClientCom/1
  MATLAB Function/2(array) -> ClientCom/3
  MATLAB Function/1(size) -> ClientCom/2
  ClientCom/1 -> Chanel State/1

== system LibrarySimulinkSASv2016a/UDP Data Server ==
  Data In : Inport
  MATLAB Function : MATLAB Function  TreatAsAtomicUnit=on
  Selector : Selector  InputPortWidth=1024  IndexOptions=Starting index (dialog)  Indices=1  OutputSizes=n
  ServerCom : S-Function  FunctionName=ServerCom  SFunctionDeploymentMode=off  EnableBusSupport=off
      mask Help:
      | This block is used to transmit information over the network.
      | This block is used to transmit small data vectors. For larger sizes use the block 'ServerComVideo'.
      | This block depends on the external program 'Servidor.exe' placed on '\SAS TOOLS\Software Network Communication V2.0.0\Data Transmission\Servidor'
      | After executing, the program is started minimized in the taskbar.
      | To initiate communication first configure the program : right click on the icon ->Configurar.
      | On section 'Configuracion de Ip y Puertos', load the input IP address and the output IP address of the computer adaptor. If there are multiple adaptors, these IP may be identical or different for the input and output data. Also select the ports for input and output.
      | On seccion 'Protocolo' select TCP or UDP. (The TCP option still in development)
      | 
      | Input Ports
      | 
      | The vector data to be transmitted must be introduced into the port 'dataSend'. Its maximun size is 1024 doubles.
      | The size of data to be transmitted must be specified on port 'sizeSend'.
      | The transmission occurs in each pulse on port 'Clock'. Use this port to set the transmission rate.
      | 
      | Output Ports
      | 
      | Port 'stateChanel'contains information concerning the transmission of data. For details of the composition of this vector right-click the icon of the taskbar -> 'Estado'
      | Port 'dataRecv' contains the data vector received from the server.
      | 
      | 
      | Shared Memory Structure:
      | 
      | typedef struct tag_NET_VIRTUAL_DEVICE_IN {
      | 	bool signalWrite;
      | 	UINT sizeMemoryOut;
      | } NET_VIRTUAL_DEVICE_IN;
      | 
      | typedef struct tag_NET_VIRTUAL_DEVICE_OUT {
      | 	bool signalRead;	//Se leen los flancos
      | 	UINT numberSentPackets;
      | 	UINT numberRecvPackets;
      | 	UINT numberLostPackets;
      | 	UINT numberDelPackets;
      | 	float rateSentPackets;
      | 	float rateRecvPackets;
      | 	float rateLostPackets;
      | 	float rateDelPackets;
      | 	float roundTripDelay;
      | 
      | 	UINT remoteNumberSentPackets;
      | 	UINT remoteNumberRecvPackets;
      | 	UINT remoteNumberLostPackets;
      | 	UINT remoteNumberDelPackets;
      | 	float remoteRateSentPackets;
      | 	float remoteRateRecvPackets;
      | 	float remoteRateLostPackets;
      | 	float remoteRateDelPackets;
      | 	float remoteRoundTripDelay;
      | } NET_VIRTUAL_DEVICE_OUT;
      | 
      | 
      | typedef struct tag_VIRTUAL_DEVICE_IN {
      | 	float dataMemory[1024];
      | } VIRTUAL_DEVICE_IN;
      | 
      | typedef struct tag_VIRTUAL_DEVICE_OUT {
      | 	float dataMemory[1024];
      | } VIRTUAL_DEVICE_OUT;
      mask DialogControl DescGroupVar Prompt: Server data transmission through the network
      mask DialogControl DescTextVar Prompt: This block is used to exchange data over a network. Use this block server-side communication.
      mask Display:
      | image('server.png')
      | color('red')
      | port_label('input',1,'clock')
      | port_label('input',2,'sizeSend')
      | port_label('input',3,'dataSend')
      | color('blue')
      | port_label('output',1,'stateChannel')
      | port_label('output',2,'dataRecv')
  Signal Generator : SignalGenerator  WaveForm=square  Frequency=1/ts
  Chanel State : Outport
  Data out : Outport  Port=2
  wires:
  ServerCom/1 -> Chanel State/1
  MATLAB Function/1(size) -> ServerCom/2
  MATLAB Function/2(array) -> ServerCom/3
  Signal Generator/1 -> ServerCom/1
  Data In/1 -> MATLAB Function/1(data)
  Selector/1 -> Data out/1
  ServerCom/2 -> Selector/1

== system LibrarySimulinkSASv2016a/V-REP Accelerometer ==
  Data Type Conversion : DataTypeConversion
  Simple Share Memory Block : S-Function  FunctionName=simpleSHM  Parameters=nombre,dispositivo,vecE,vecS,tipoE,tipoS  SFunctionDeploymentMode=off  EnableBusSupport=off  mask(Share Memory Block version 2.0){nombre='VREP_accelerometerData', dispositivo=n, vecE=[], vecS=[3], tipoE=[], tipoS=['f']}
      mask prompts: nombre "Share Memory base name"; dispositivo "Device Number"; vecE "Sizes input vector"; vecS "Sizes output vector"; tipoE "Data Types Inputs"; tipoS "Data Types Outputs"
      mask help: This block allows Simulink communication with external processes through shared memory. It works on Unix and Windows
      mask Help:
      | Share Memory base name (nombre): It's the base string of share memory ej 'robot'
      | Device Number (dispositivo): integer that identifies the device between multiple in use
      | Only one Share memory will be created, so you should specify only the inputs or outputs but not both
      | One shared memorys is created with name: "%name_%dispositivo"
      | 
      | Sizes input vector (vecE)  : array that specify the number of inputs (array dimension) and the inputs size (array values)
      | Sizes output vector  (vecS): array that specify the number of outputs (array dimension) and the outputs size (array values)
      | Data types input(tipoE): char array that specify each input types
      | Data types output (tipoS): char array that specify each output types
      | Allowed types are:
      | ('f'=float, 'd'=double, 'i'=int32, 'c'=char, 'C'= unsigned char, 'I'=unsigned int)
      | 
      | Example.
      | Suppose the following parameters:
      | nombre='exampleMemory'
      | dispositivo=10
      | vecE=[3 2 1]
      | vecS=[]
      | tipoE=['f' 'I' 'c']
      | tipoS=[]
      | 
      | One Share Memorie is created with name:
      | "exampleMemory_10" (block input)
      | 
      | 3 inputs are created:
      |  1° Dimension 3, type "unsigned char"
      |  2° Dimension 2, type "int"
      |  3° Dimension 1, type "char"
      mask Display: image('memoryChip.png')
  Acceleration Data : Outport
  wires:
  Data Type Conversion/1 -> Acceleration Data/1
  Simple Share Memory Block/1 -> Data Type Conversion/1
